simulink subsystem "ButtonStatesRF"  (R2023b) (continued)
== stateflow chart ButtonStatesRF/ButtonStates/buttonFlowChart == (continued)
                  Flow.ButtonTriggeredMenu.Menu.MainSubMenu.SettingsSubMenu.ClockSubMenu.DateSubMenu.DateYYYYMMDD.DateDigit3 -> Flow.ButtonTriggeredMenu.Menu.MainSubMenu.SettingsSubMenu.ClockSubMenu.DateSubMenu.DateYYYYMMDD.DateDigit2 [order 1] : EXIT
                  (junction 7832) -> 7840
                  (junction 7837) -> (junction 7832) : {BLU_FnClkSaveDate();}
                  Flow.ButtonTriggeredMenu.Menu.MainSubMenu.SettingsSubMenu.ClockSubMenu.DateSubMenu.DateYYYYMMDD.DateDigit3 -> (junction 7833) [order 6] : longUP
                      | {BLU_FnClkIncDateDig1();}
                  (junction 7833) -> (junction 7834)
                  Flow.ButtonTriggeredMenu.Menu.MainSubMenu.SettingsSubMenu.ClockSubMenu.DateSubMenu.DateYYYYMMDD.DateDigit3 -> (junction 7834) [order 4] : UP
                      | {BLU_FnClkIncDateDig1();}
                  (junction 7834) -> (junction 7835)
                  Flow.ButtonTriggeredMenu.Menu.MainSubMenu.SettingsSubMenu.ClockSubMenu.DateSubMenu.DateYYYYMMDD.DateDigit3 -> (junction 7835) [order 3] : DOWN
                      | {BLU_FnClkDecDateDig1();}
                  (junction 7835) -> (junction 7836)
                  Flow.ButtonTriggeredMenu.Menu.MainSubMenu.SettingsSubMenu.ClockSubMenu.DateSubMenu.DateYYYYMMDD.DateDigit3 -> (junction 7836) [order 2] : longDOWN
                      | {BLU_FnClkDecDateDig1();}
                  (junction 7836) -> (junction 7838)
                  Flow.ButtonTriggeredMenu.Menu.MainSubMenu.SettingsSubMenu.ClockSubMenu.DateSubMenu.DateYYYYMMDD.DateDigit3 -> (junction 7837) [order 5] : SET
                  (junction 7838) -> Flow.ButtonTriggeredMenu.Menu.MainSubMenu.SettingsSubMenu.ClockSubMenu.DateSubMenu.DateYYYYMMDD.DateDigit3
                7679 -> (junction 7674)
                (junction 7674) -> 7680 [order 1] : [dateFormat == eStateName.DATE_FORMAT_DDMMYYYY]
                (junction 7674) -> (junction 7676) [order 2]
                7681 -> (junction 7675)
                (junction 7675) -> (junction 7677)
                (junction 7676) -> 7682 [order 1] : [dateFormat == eStateName.DATE_FORMAT_MMDDYYYY]
                (junction 7676) -> (junction 7678) [order 2]
                7683 -> (junction 7677)
                (junction 7677) -> 7686
                (junction 7678) -> 7684
                7685 -> 7686
              state SetClockFormat
                (history)
                state SetClockFormatMenu
                  state Clock12H
                    en:
                    currentState = eStateName.CLOCK_FORMAT_12H;
                  state Clock24H
                    en:
                    currentState = eStateName.CLOCK_FORMAT_24H;
                  Clock12H -> Clock24H : UP || DOWN
                  Clock24H -> Clock12H : UP || DOWN
                7884 -> Clock12H
                SetClockFormatMenu -> 7885 [order 1] : EXIT
                (junction 7883) -> 7885
                SetClockFormatMenu -> (junction 7883) [order 2] : SET
                    | {BLU_FnClkFrmtTgle(currentState);}
              state SetDateFormat
                (history)
                state SetDateFormatMenu
                  (history)
                  state DateFormat1
                    en:
                    currentState = eStateName.DATE_FORMAT_DDMMYYYY;
                  state DateFormat2
                    en:
                    currentState = eStateName.DATE_FORMAT_MMDDYYYY;
                  state DateFormat3
                    en:
                    currentState = eStateName.DATE_FORMAT_YYYYMMDD;
                  (default) -> DateFormat1
                  (junction 7905) -> DateFormat1
                  DateFormat1 -> (junction 7906) [order 2] : UP
                  (junction 7907) -> (junction 7905)
                  (junction 7906) -> (junction 7908)
                  DateFormat1 -> DateFormat2 [order 1] : DOWN
                  DateFormat2 -> DateFormat1 [order 2] : UP
                  DateFormat2 -> DateFormat3 [order 1] : DOWN
                  DateFormat3 -> DateFormat2 [order 2] : UP
                  DateFormat3 -> (junction 7907) [order 1] : DOWN
                  (junction 7908) -> DateFormat3
                7897 -> DateFormat1
                SetDateFormatMenu -> 7898 [order 1] : EXIT
                (junction 7896) -> 7898 : {dateFormat =currentState;
                    | BLU_FnDateFrmtTgle(currentState);}
                SetDateFormatMenu -> (junction 7896) [order 2] : SET
              (default) -> ClockDateMenu
              7582 -> (junction 7612)
              (junction 7613) -> 7583 [order 1] : SET
              7584 -> (junction 7577)
              (junction 7577) -> (junction 7578)
              (junction 7579) -> 7585
              Date -> 7586 [order 3] : SET
              7587 -> (junction 7578)
              ClockDateMenu -> (junction 7579) : EXIT
              (junction 7578) -> (junction 7580)
              (junction 7579) -> 7588 [commented out] : [IgnBattFlg==true]
              ClockFormat -> 7589 [order 3] : SET
              7590 -> (junction 7580)
              (junction 7580) -> (junction 7581)
              DateFormat -> 7591 [order 3] : SET
              (junction 7581) -> ClockDateMenu
              7592 -> (junction 7581)
            state UnitsSubMenu
              (history)
              state UnitsMenu
                (history)
                state Distance
                  en:
                  currentState = eStateName.DISTANCE;
                state Temperature
                  en:
                  currentState = eStateName.TEMPERATURE;
                state Consumption
                  en:
                  currentState = eStateName.CONSUMPTION;
                (default) -> Distance
                (junction 7942) -> Distance
                (junction 7945) -> (junction 7942)
                Distance -> (junction 7943) [order 3] : UP
                (junction 7943) -> (junction 7944)
                Distance -> Temperature [order 1] : DOWN
                Temperature -> Distance [order 1] : UP
                Temperature -> Consumption [order 2] : DOWN
                Consumption -> Temperature [order 1] : UP
                (junction 7944) -> Consumption
                Consumption -> (junction 7945) [order 3] : DOWN
              state DistanceSubMenu
                state DstnceMenu
                  (history)
                  state Kilometer
                    en:
                    currentState = eStateName.DISTANCE_KILOMETER;
                  state Miles
                    en:
                    currentState = eStateName.DISTANCE_MILES;
                  (default) -> Kilometer
                  Kilometer -> Miles : DOWN || UP
                  Miles -> Kilometer : UP || DOWN
                7962 -> DstnceMenu
                DstnceMenu -> 7963 [order 1] : EXIT
                (junction 7961) -> 7963 : {BLU_FnSETDistUnits(currentState);}
                DstnceMenu -> (junction 7961) [order 2] : SET
              state TemperatureSubMenu
                state TempUnitsMenu
                  (history)
                  state Celsius
                    en:
                    currentState = eStateName.TEMPERATURE_CELSIUS;
                  state Farenheit
                    en:
                    currentState = eStateName.TEMPERATURE_FARENHEIT;
                  (default) -> Celsius
                  Celsius -> Farenheit : DOWN || UP
                  Farenheit -> Celsius : UP || DOWN
                7977 -> TempUnitsMenu
                TempUnitsMenu -> (junction 7976) [order 2] : SET
                (junction 7976) -> 7978 : {BLU_FnSETTempUnits(currentState);}
                TempUnitsMenu -> 7978 [order 1] : EXIT
              state ConsumptionSubMenu
                state ConsUnits
                  (history)
                  state litres_100km
                    en:
                    currentState = eStateName.CONSUMPTION_LTR_PER_100KM;
                  state km_l
                    en:
                    currentState = eStateName.CONSUMPTION_KM_PER_LTR;
                  state USg_100mile
                    en:
                    currentState = eStateName.CONSUMPTION_USG_PER_100MI;
                  state Mi_USg
                    en:
                    currentState = eStateName.CONSUMPTION_MI_PER_USG;
                  state Mi_Litre
                    en:
                    currentState = eStateName.CONSUMPTION_MI_PER_LTR;
                  state UKg_100Mi
                    en:
                    currentState = eStateName.CONSUMPTION_UKG_PER_100MI;
                  state Mi_UKg
                    en:
                    currentState = eStateName.CONSUMPTION_MI_PER_UKG;
                  state Li_100Mi
                    en:
                    currentState = eStateName.CONSUMPTION_L_PER_100MI;
                  (default) -> litres_100km
                  litres_100km -> (junction 8000) [order 2] : UP
                  (junction 8001) -> litres_100km
                  (junction 8000) -> (junction 8003)
                  (junction 8002) -> (junction 8001)
                  litres_100km -> km_l [order 1] : DOWN
                  km_l -> litres_100km [order 1] : UP
                  km_l -> USg_100mile [order 2] : DOWN
                  USg_100mile -> km_l [order 1] : UP
                  USg_100mile -> Mi_USg [order 2] : DOWN
                  Mi_USg -> USg_100mile [order 1] : UP
                  Mi_USg -> Mi_Litre [order 2] : DOWN
                  Mi_Litre -> Mi_USg [order 1] : UP
                  Mi_Litre -> UKg_100Mi [order 2] : DOWN
                  UKg_100Mi -> Mi_Litre [order 1] : UP
                  UKg_100Mi -> Mi_UKg [order 2] : DOWN
                  Mi_UKg -> UKg_100Mi [order 1] : UP
                  Mi_UKg -> Li_100Mi [order 2] : DOWN
                  Li_100Mi -> Mi_UKg [order 1] : UP
                  Li_100Mi -> (junction 8002) [order 2] : DOWN
                  (junction 8003) -> Li_100Mi
                7992 -> ConsUnits
                ConsUnits -> 7993 [order 1] : EXIT
                (junction 7991) -> 7993 : {BLU_FnSETConsUnits(currentState);}
                ConsUnits -> (junction 7991) [order 2] : SET
              7924 -> UnitsMenu
              Distance -> 7925 [order 2] : SET
              7926 -> UnitsMenu
              UnitsMenu -> 7927 : EXIT
              Temperature -> 7928 [order 3] : SET
              7929 -> UnitsMenu
              Consumption -> 7930 [order 2] : SET
              7931 -> UnitsMenu
            state LanguageSubMenu
              (history)
              state LanguageSetSubMenu
                (history)
                state EnglishUS
                  en:
                  currentState = eStateName.ENGLISH_US;
                state EnglishUK
                  en:
                  currentState = eStateName.ENGLISH_UK;
                state German
                  en:
                  currentState = eStateName.GERMAN;
                state Italian
                  en:
                  currentState = eStateName.ITALIAN;
                state French
                  en:
                  currentState = eStateName.FRENCH;
                state Spanish
                  en:
                  currentState = eStateName.SPANISH;
                (junction 8043) -> EnglishUS
                (junction 8046) -> (junction 8043)
                EnglishUS -> (junction 8044) [order 2] : UP
                (junction 8044) -> (junction 8045)
                EnglishUS -> EnglishUK [order 1] : DOWN
                EnglishUK -> EnglishUS [order 1] : UP
                EnglishUK -> German [order 2] : DOWN
                German -> EnglishUK [order 1] : UP
                German -> Italian [order 2] : DOWN
                Italian -> German [order 1] : UP
                Italian -> French [order 2] : DOWN
                French -> Italian [order 1] : UP
                French -> Spanish [order 2] : DOWN
                Spanish -> French [order 1] : UP
                (junction 8045) -> Spanish
                Spanish -> (junction 8046) [order 2] : DOWN
              8035 -> (junction 8043)
              LanguageSetSubMenu -> 8036 [order 1] : EXIT
              (junction 8034) -> 8036 : {BLU_FnSETLanguage(currentState);}
              LanguageSetSubMenu -> (junction 8034) [order 2] : SET
            state HeatingGripsSettingsSubMenu
              (history)
              state HtdGripsSel
                (history)
                state HeatingGripsNotAvailable
                  en:
                  currentState = eStateName.HEATING_GRIPS_NOT_AVAILABLE;
                state HeatingGripsAvailable
                  en:
                  currentState = eStateName.HEATING_GRIPS_AVAILABLE;
                HeatingGripsNotAvailable -> HeatingGripsAvailable [order 1] : DOWN || UP
                HeatingGripsAvailable -> HeatingGripsNotAvailable [order 1] : DOWN || UP
                HeatingGripsAvailable -> HeatingGripsAvailable [commented out] : SET
                    | {heatedGrips = eFeatureStatus.available;
                    | BLU_FnSetHeatedGrips(heatedGrips);}
                    | //TBD Heated_Grips variable set
              state HCU_GripHeat_LvlChk
                en:
                currentState = eStateName.HCU_GRIP_HEAT_LVL_CHK;
              HeatingGripsNotAvailable -> 8072 [order 2] : SET
                  | {heatedGrips = eFeatureStatus.notAvailable;
                  | DASH_Grip_Installation = 0;
                  | BLU_FnSetHeatedGrips(heatedGrips);}
                  | //TBD Heated_Grips variable set
              (junction 8070) -> 8072
              8073 -> HeatingGripsNotAvailable
              HtdGripsSel -> (junction 8070) : EXIT
              (junction 8071) -> (junction 8070) : [HCU_Grip_Heat_Level_Res ==0 || after(3,sec)]
              HCU_GripHeat_LvlChk -> (junction 8070) [order 1] : [HCU_Grip_Heat_Level_Res !=0]
                  | {heatedGrips = eFeatureStatus.notAvailable;}
              HCU_GripHeat_LvlChk -> (junction 8071) [order 2]
              HeatingGripsAvailable -> HCU_GripHeat_LvlChk [order 2] : SET
                  | {heatedGrips = eFeatureStatus.available;
                  | DASH_Grip_Installation = 1;}
                  | //TBD Heated_Grips variable set
            state ExtraFunctionsSubMenu
              %en:
              %currentState = eStateName.EXTRA_FUNCTIONS_SUB_MN;
              state ExtraFncSubMenu
                state ExtraFnCall
                  en:
                  currentState = eStateName.EXTRA_FN_CALL;
                state ExtraFnAudio
                  en:
                  currentState = eStateName.EXTRA_FN_AUDIO;
                state ExtraFnNavigation
                  en:
                  currentState = eStateName.EXTRA_FN_NAVIGATION;
                state ExtraFnRemoteCtrl
                  en:
                  currentState = eStateName.EXTRA_FN_REMOTE_CTRL;
                (default) -> Flow.ButtonTriggeredMenu.Menu.MainSubMenu.SettingsSubMenu.ExtraFunctionsSubMenu.ExtraFncSubMenu.ExtraFnCall
                Flow.ButtonTriggeredMenu.Menu.MainSubMenu.SettingsSubMenu.ExtraFunctionsSubMenu.ExtraFncSubMenu.ExtraFnCall -> 8337 [order 3] : SET
                (junction 8333) -> Flow.ButtonTriggeredMenu.Menu.MainSubMenu.SettingsSubMenu.ExtraFunctionsSubMenu.ExtraFncSubMenu.ExtraFnCall
                (junction 8331) -> (junction 8333)
                Flow.ButtonTriggeredMenu.Menu.MainSubMenu.SettingsSubMenu.ExtraFunctionsSubMenu.ExtraFncSubMenu.ExtraFnCall -> (junction 8324) [order 2] : UP
                (junction 8324) -> (junction 8326)
                Flow.ButtonTriggeredMenu.Menu.MainSubMenu.SettingsSubMenu.ExtraFunctionsSubMenu.ExtraFncSubMenu.ExtraFnCall -> Flow.ButtonTriggeredMenu.Menu.MainSubMenu.SettingsSubMenu.ExtraFunctionsSubMenu.ExtraFncSubMenu.ExtraFnAudio [order 1] : DOWN
                Flow.ButtonTriggeredMenu.Menu.MainSubMenu.SettingsSubMenu.ExtraFunctionsSubMenu.ExtraFncSubMenu.ExtraFnAudio -> Flow.ButtonTriggeredMenu.Menu.MainSubMenu.SettingsSubMenu.ExtraFunctionsSubMenu.ExtraFncSubMenu.ExtraFnCall [order 1] : UP
                Flow.ButtonTriggeredMenu.Menu.MainSubMenu.SettingsSubMenu.ExtraFunctionsSubMenu.ExtraFncSubMenu.ExtraFnAudio -> 8340 [order 3] : SET
                Flow.ButtonTriggeredMenu.Menu.MainSubMenu.SettingsSubMenu.ExtraFunctionsSubMenu.ExtraFncSubMenu.ExtraFnAudio -> Flow.ButtonTriggeredMenu.Menu.MainSubMenu.SettingsSubMenu.ExtraFunctionsSubMenu.ExtraFncSubMenu.ExtraFnNavigation [order 2] : DOWN
                Flow.ButtonTriggeredMenu.Menu.MainSubMenu.SettingsSubMenu.ExtraFunctionsSubMenu.ExtraFncSubMenu.ExtraFnNavigation -> Flow.ButtonTriggeredMenu.Menu.MainSubMenu.SettingsSubMenu.ExtraFunctionsSubMenu.ExtraFncSubMenu.ExtraFnAudio [order 1] : UP
                Flow.ButtonTriggeredMenu.Menu.MainSubMenu.SettingsSubMenu.ExtraFunctionsSubMenu.ExtraFncSubMenu.ExtraFnNavigation -> 8343 [order 3] : SET
                Flow.ButtonTriggeredMenu.Menu.MainSubMenu.SettingsSubMenu.ExtraFunctionsSubMenu.ExtraFncSubMenu.ExtraFnNavigation -> Flow.ButtonTriggeredMenu.Menu.MainSubMenu.SettingsSubMenu.ExtraFunctionsSubMenu.ExtraFncSubMenu.ExtraFnRemoteCtrl [order 2] : DOWN
                Flow.ButtonTriggeredMenu.Menu.MainSubMenu.SettingsSubMenu.ExtraFunctionsSubMenu.ExtraFncSubMenu.ExtraFnRemoteCtrl -> Flow.ButtonTriggeredMenu.Menu.MainSubMenu.SettingsSubMenu.ExtraFunctionsSubMenu.ExtraFncSubMenu.ExtraFnNavigation [order 1] : UP
                (junction 8326) -> Flow.ButtonTriggeredMenu.Menu.MainSubMenu.SettingsSubMenu.ExtraFunctionsSubMenu.ExtraFncSubMenu.ExtraFnRemoteCtrl
                Flow.ButtonTriggeredMenu.Menu.MainSubMenu.SettingsSubMenu.ExtraFunctionsSubMenu.ExtraFncSubMenu.ExtraFnRemoteCtrl -> 8345 [order 3] : SET
                Flow.ButtonTriggeredMenu.Menu.MainSubMenu.SettingsSubMenu.ExtraFunctionsSubMenu.ExtraFncSubMenu.ExtraFnRemoteCtrl -> (junction 8331) [order 2] : DOWN
              state ExtraFnCall
                en:
                currentState = eStateName.EXTRA_FN_CALL_WAIT;
              state ExtraFnAudio
                en:
                currentState = eStateName.EXTRA_FN_AUDIO_WAIT;
              state ExtraFnNavigation
                en:
                currentState = eStateName.EXTRA_FN_NAVIGATION_WAIT;
              state ExtraFnRemoteCtrl
                en:
                currentState = eStateName.EXTRA_FN_REMOTE_CTRL_WAIT;
              (default) -> ExtraFncSubMenu
              8338 -> Flow.ButtonTriggeredMenu.Menu.MainSubMenu.SettingsSubMenu.ExtraFunctionsSubMenu.ExtraFnCall
              Flow.ButtonTriggeredMenu.Menu.MainSubMenu.SettingsSubMenu.ExtraFunctionsSubMenu.ExtraFnCall -> (junction 8359)
              (junction 8359) -> (junction 8361)
              8342 -> Flow.ButtonTriggeredMenu.Menu.MainSubMenu.SettingsSubMenu.ExtraFunctionsSubMenu.ExtraFnAudio
              Flow.ButtonTriggeredMenu.Menu.MainSubMenu.SettingsSubMenu.ExtraFunctionsSubMenu.ExtraFnAudio -> (junction 8361)
              (junction 8361) -> (junction 8364)
              8347 -> Flow.ButtonTriggeredMenu.Menu.MainSubMenu.SettingsSubMenu.ExtraFunctionsSubMenu.ExtraFnNavigation
              Flow.ButtonTriggeredMenu.Menu.MainSubMenu.SettingsSubMenu.ExtraFunctionsSubMenu.ExtraFnNavigation -> (junction 8364)
              (junction 8364) -> (junction 8367)
              8348 -> Flow.ButtonTriggeredMenu.Menu.MainSubMenu.SettingsSubMenu.ExtraFunctionsSubMenu.ExtraFnRemoteCtrl
              Flow.ButtonTriggeredMenu.Menu.MainSubMenu.SettingsSubMenu.ExtraFunctionsSubMenu.ExtraFnRemoteCtrl -> (junction 8367)
              (junction 8367) -> (junction 8370)
              (junction 8370) -> ExtraFncSubMenu : [after(BLU_ExtraFnWtTmr_Tms,msec)]
              ExtraFncSubMenu -> 8375 : EXIT
            6185 -> 6186
            6187 -> 6188
            6189 -> (junction 6255)
            6191 -> 6190
            6192 -> 6193
            SettingsMenuScreens -> 6194 : EXIT
            6196 -> 6195
            6197 -> 6198
            6199 -> 6200
            6202 -> 6201
            6203 -> 6204
            6206 -> 6205
            6207 -> ClockSubMenu
            6208 -> 6211 [commented out]
            6209 -> 6210
            6213 -> 6212
            6214 -> 6215
            6217 -> 6216
            6218 -> 6219
            6221 -> 6220
            6222 -> 6223
            6225 -> 6224
            6226 -> ExtraFunctionsSubMenu
            8376 -> 6227
          state SetService
            (history)
            state ServiceMenu
              (history)
              state SetServiceDistance
                state SetServiceDistanceKm
                  en:
                  currentState = eStateName.SET_SERVICE_DISTANCE_KM;
                state SetServiceDistanceMi
                  en:
                  currentState = eStateName.SET_SERVICE_DISTANCE_MI;
                (default) -> (junction 8152)
                (junction 8152) -> Flow.ButtonTriggeredMenu.Menu.MainSubMenu.SetService.ServiceMenu.SetServiceDistance.SetServiceDistanceKm [order 1] : [odoUnits == 0]
                (junction 8152) -> (junction 8153) [order 2]
                (junction 8153) -> Flow.ButtonTriggeredMenu.Menu.MainSubMenu.SetService.ServiceMenu.SetServiceDistance.SetServiceDistanceMi
              state SetServiceMonths
                en:
                currentState = eStateName.SET_SERVICE_MONTHS_MM;
              state SoftwareVersion
                en:
                currentState = eStateName.SET_SERVICE_SOFTWARE_VERSION;
              (default) -> SetServiceDistance
              SetServiceDistance -> (junction 8385) [order 3] : UP
              (junction 8385) -> (junction 8387)
              (junction 8392) -> SetServiceDistance
              (junction 8397) -> (junction 8392)
              SetServiceDistance -> SetServiceMonths [order 2] : DOWN
              SetServiceMonths -> SetServiceDistance [order 2] : UP
              SetServiceMonths -> SoftwareVersion [order 3] : DOWN
              SoftwareVersion -> SetServiceMonths [order 1] : UP
              (junction 8387) -> SoftwareVersion
              SoftwareVersion -> (junction 8397) [order 2] : DOWN
            state SetServiceDistanceMenu
              (history)
              state DistanceModifyMenu
                (history)
                state SetServiceDistanceKm
                  en:
                  currentState = eStateName.CURSOR_ON_KM;
                state SetServiceDistanceMi
                  en:
                  currentState = eStateName.CURSOR_ON_MI;
              (default) -> (junction 8163)
              Flow.ButtonTriggeredMenu.Menu.MainSubMenu.SetService.SetServiceDistanceMenu.DistanceModifyMenu.SetServiceDistanceKm -> (junction 8162) [order 2] : DOWN
              (junction 8162) -> (junction 8166) : {BLU_FnDecSrvcDistKM();}
              (junction 8163) -> Flow.ButtonTriggeredMenu.Menu.MainSubMenu.SetService.SetServiceDistanceMenu.DistanceModifyMenu.SetServiceDistanceKm [order 1] : [odoUnits == 0]
              Flow.ButtonTriggeredMenu.Menu.MainSubMenu.SetService.SetServiceDistanceMenu.DistanceModifyMenu.SetServiceDistanceKm -> (junction 8164) [order 1] : UP
              (junction 8163) -> (junction 8168) [order 2]
              (junction 8164) -> (junction 8165) : {BLU_FnIncSrvcDistKM();}
              (junction 8165) -> DistanceModifyMenu
              (junction 8166) -> (junction 8165)
              (junction 8167) -> (junction 8165) : {BLU_FnIncSrvcDistMI();}
              (junction 8169) -> (junction 8166) : {BLU_FnDecSrvcDistMI();}
              Flow.ButtonTriggeredMenu.Menu.MainSubMenu.SetService.SetServiceDistanceMenu.DistanceModifyMenu.SetServiceDistanceMi -> (junction 8167) [order 1] : UP
              (junction 8168) -> Flow.ButtonTriggeredMenu.Menu.MainSubMenu.SetService.SetServiceDistanceMenu.DistanceModifyMenu.SetServiceDistanceMi
              Flow.ButtonTriggeredMenu.Menu.MainSubMenu.SetService.SetServiceDistanceMenu.DistanceModifyMenu.SetServiceDistanceMi -> (junction 8169) [order 2] : DOWN
            state SetServiceMonthsMenu
              en:
              currentState = eStateName.CURSON_ON_DATE;
            (default) -> ServiceMenu
            ServiceMenu -> (junction 8123) [order 2] : EXIT
            (junction 8123) -> 8129
            ServiceMenu -> 8129 [order 1] : [duration(set,msec) >=3000]
                | {BLU_FnSaveSrvcChanges();}
            SetServiceDistance -> SetServiceDistanceMenu [order 1] : SET
            SetServiceDistanceMenu -> (junction 8124) : EXIT || SET
            (junction 8124) -> ServiceMenu
            SetServiceMonthsMenu -> (junction 8124) [order 3] : EXIT || SET
            SetServiceMonthsMenu -> (junction 8125) [order 1] : UP
            (junction 8125) -> (junction 8126) : {BLU_FnIncSrvcMonths();}
            SetServiceMonths -> SetServiceMonthsMenu [order 1] : SET
            (junction 8126) -> SetServiceMonthsMenu
            (junction 8128) -> (junction 8126)
            SetServiceMonthsMenu -> (junction 8127) [order 2] : DOWN
            (junction 8127) -> (junction 8128) : {BLU_FnDecSrvcMonths();}
          (default) -> (junction 3141)
          (junction 8255) -> 8260 [order 2] : EXIT
          5276 -> (junction 3141)
          (junction 3141) -> LapTimer [order 1] : [rideMode == eRideMode.track && ...
              | variant !=eBikeVariant.duke200]
          (junction 3141) -> Motorcycle [order 2]
          (junction 3145) -> (junction 3141)
          SettingsMenu -> (junction 8193) [order 2]
          (junction 8255) -> 8199 [order 1] : SET
          (junction 8091) -> (junction 8255) [order 2]
          LapTimer -> (junction 3151) [order 2] : UP
          8198 -> LapTimer
          (junction 4100) -> (junction 3151) [order 2]
          (junction 3151) -> (junction 3153)
          (junction 4100) -> LapTimer [order 1] : [rideMode == eRideMode.track && ...
              | variant !=eBikeVariant.duke200]
          LapTimer -> Motorcycle [order 1] : DOWN
          LapTimerEntry -> (junction 8255)
          Motorcycle -> (junction 4100) [order 1] : UP
          NavigationVisible -> (junction 8100)
          5941 -> 5671
          Motorcycle -> (junction 8091) [order 3]
          (junction 8091) -> 5672 [order 1] : SET
          (junction 8093) -> (junction 8091) [order 2]
          5944 -> 5670
          5673 -> Motorcycle
          5947 -> 5669
          Motorcycle -> BikeInfo [order 2] : DOWN
          BikeInfo -> Motorcycle [order 1] : UP
          5568 -> 5520
          BikeInfo -> (junction 8093) [order 3]
          (junction 8093) -> 5518 [order 1] : SET
          (junction 8095) -> (junction 8093) [order 2]
          5519 -> BikeInfo
          BikeInfo -> TripInfo [order 2] : DOWN
          TripInfo -> BikeInfo [order 1] : UP
          AudioVisible -> (junction 8107)
          (junction 8095) -> 5573 [order 1] : SET
          TripInfo -> (junction 8095) [order 3]
          5664 -> 5570
          (junction 8097) -> (junction 8095) [order 2]
          (junction 839) -> TripInfo
          5572 -> (junction 839)
          5666 -> 5571
          CruiseControlMain -> (junction 839) [order 1] : UP
          (junction 194) -> (junction 839) [order 2]
          TripInfo -> (junction 154) [order 2] : DOWN
          6030 -> 5950
          CruiseControlMain -> (junction 8097) [order 3]
          (junction 8097) -> 5952 [order 1] : SET
          (junction 8100) -> (junction 8097) [order 2]
          (junction 154) -> CruiseControlMain [order 1] : [variant !=eBikeVariant.duke200 && ...
              | (ECU_SL_Feature == 1 || ...
              | rideMode != eRideMode.track)]
          5951 -> (junction 194)
          (junction 194) -> CruiseControlMain [order 1] : [ECU_SL_Feature == 1 && ...
              | variant !=eBikeVariant.duke200]
          NavigationMain -> (junction 194) [order 2] : UP
          (junction 154) -> (junction 157) [order 2]
          (junction 189) -> (junction 194) [order 2]
          CruiseControlMain -> (junction 157) [order 2] : DOWN
          6085 -> 6033
          (junction 8100) -> 6035 [order 1] : SET
          (junction 8103) -> (junction 8100) [order 2]
          (junction 157) -> NavigationMain [order 1] : [CCU_Status == eCCUStatus.installed &&...
              |  CCU_TbT_Navigation_Feature == 1 &&...
              |  btPhonePrngScs ]
          (junction 189) -> NavigationMain [order 1] : [CCU_Status == eCCUStatus.installed &&...
              |  CCU_TbT_Navigation_Feature == 1 &&...
              | btPhonePrngScs]
          6034 -> (junction 189)
          (junction 157) -> (junction 163) [order 2]
          HeatedGripsMain -> (junction 189) [order 2] : UP
          (junction 186) -> (junction 189) [order 2]
          NavigationMain -> (junction 163) [order 1] : DOWN
          HeatedGripsMain -> (junction 8103) [order 3]
          (junction 8103) -> 6089 [order 1] : SET
          6123 -> 6088
          (junction 8107) -> (junction 8103) [order 2]
          (junction 163) -> HeatedGripsMain [order 1] : [variant !=eBikeVariant.duke200 && ...
              | Heated_Grips == 1 ]
              |  //Menu Settings/Heating/Heating Grip == Not available]
          (junction 163) -> (junction 171) [order 2]
          (junction 186) -> HeatedGripsMain [order 1] : [Heated_Grips == 1 &&...
              | variant !=eBikeVariant.duke200 ]
          6090 -> (junction 186)
          Flow.ButtonTriggeredMenu.Menu.MainSubMenu.AudioMain -> (junction 186) [order 2] : UP
          (junction 183) -> (junction 186) [order 2] : %[CCU_Status == eCCUStatus.notInstalled ||...
              | % CCU_Music_Feature == 0]
          HeatedGripsMain -> (junction 171) [order 1] : DOWN
          6167 -> 6126
          (junction 8107) -> 6128 [order 1] : SET
          (junction 8193) -> (junction 8107) [order 2]
          (junction 171) -> Flow.ButtonTriggeredMenu.Menu.MainSubMenu.AudioMain [order 1] : [CCU_Status == eCCUStatus.installed &&...
              |  CCU_Music_Feature == 1 &&...
              | btPhonePrngScs]
          (junction 171) -> (junction 177) [order 2]
          6127 -> (junction 183)
          (junction 183) -> Flow.ButtonTriggeredMenu.Menu.MainSubMenu.AudioMain [order 1] : [CCU_Status == eCCUStatus.installed &&...
              |  CCU_Music_Feature == 1 &&...
              | btPhonePrngScs]
          (junction 5313) -> (junction 183)
          (junction 181) -> (junction 183)
          Flow.ButtonTriggeredMenu.Menu.MainSubMenu.AudioMain -> (junction 177) [order 1] : DOWN
          (junction 3153) -> (junction 4104)
          (junction 4104) -> (junction 5313) [order 2]
          (junction 8307) -> (junction 5313)
          (junction 4104) -> SettingsMain [order 1] : [vehSpeed<2.5]
          SettingsMain -> (junction 5313) [order 3] : [vehSpeed>3.0]
          SettingsSubMenu -> (junction 8307) : [vehSpeed>3.0]
          SettingsMain -> (junction 181) [order 1] : UP
          (junction 8193) -> 6179 [order 1] : SET
          8118 -> 6181
          (junction 177) -> SettingsMain [order 1] : [vehSpeed<=2.5]
          (junction 177) -> (junction 3145) [order 2]
          (junction 8189) -> SettingsMain
          6180 -> (junction 8189)
          8121 -> (junction 8189)
          SettingsMain -> (junction 3145) [order 2] : DOWN
          3012 -> SetService
        state Lap_TimerV1 [commented out]
          state LapTimerLoop [commented out]
            en:
            currentState = eStateName.RESET_LAPS;
            roundTimer = roundTimerData_arr[roundTimeCounter];
          state ResetLaps [commented out]
            state Keep [commented out]
              en:
              currentState = eStateName.KEEP;
            state Reset [commented out]
              en:
              currentState = eStateName.RESET;
              roundTimerData_arr[roundTimeCounter] =0;
              //updateRoundTimeData();
            (default) -> Flow.ButtonTriggeredMenu.Menu.Lap_TimerV1.ResetLaps.Keep [commented out]
            Flow.ButtonTriggeredMenu.Menu.Lap_TimerV1.ResetLaps.Keep -> Flow.ButtonTriggeredMenu.Menu.Lap_TimerV1.ResetLaps.Reset [commented out] : DOWN
            Flow.ButtonTriggeredMenu.Menu.Lap_TimerV1.ResetLaps.Reset -> Flow.ButtonTriggeredMenu.Menu.Lap_TimerV1.ResetLaps.Keep [commented out] : UP
          (default) -> Flow.ButtonTriggeredMenu.Menu.Lap_TimerV1.LapTimerLoop [commented out] : {roundTimeCounter = 0;
              | copyRoundTimeData();
              | updateRoundTimeData();}
          Flow.ButtonTriggeredMenu.Menu.Lap_TimerV1.LapTimerLoop -> (junction 237) [commented out] : DOWN
          (junction 237) -> (junction 240) [commented out] : [roundTimeCounter < BLU_MaxRndTimeLoopLim]
          (junction 240) -> (junction 239) [commented out] : {roundTimeCounter++;}
          (junction 237) -> (junction 239) [commented out]
          (junction 239) -> Flow.ButtonTriggeredMenu.Menu.Lap_TimerV1.LapTimerLoop [commented out]
          Flow.ButtonTriggeredMenu.Menu.Lap_TimerV1.LapTimerLoop -> (junction 245) [commented out] : UP
          (junction 245) -> (junction 247) [commented out] : [roundTimeCounter>0]
          (junction 247) -> (junction 249) [commented out] : {roundTimeCounter--;}
          (junction 245) -> (junction 249) [commented out]
          (junction 249) -> Flow.ButtonTriggeredMenu.Menu.Lap_TimerV1.LapTimerLoop [commented out]
          Flow.ButtonTriggeredMenu.Menu.Lap_TimerV1.LapTimerLoop -> Flow.ButtonTriggeredMenu.Menu.Lap_TimerV1.ResetLaps [commented out] : SET
          Flow.ButtonTriggeredMenu.Menu.Lap_TimerV1.ResetLaps -> Flow.ButtonTriggeredMenu.Menu.Lap_TimerV1.LapTimerLoop [commented out] : EXIT
        state NoQS
          en:
          QuickSelectorDbg = 1;
        (junction 2598) -> 2564
        4635 -> 2564
        NoQS -> (junction 2598) : [after(2,sec)]
        (junction 2147) -> (junction 2234) [order 1] : [quickSelectorUp ==eStateName.QS_UP_RIDE_MODE && ...
            | variant !=eBikeVariant.duke200]
        (junction 2147) -> NoQS [order 2]
        (junction 2425) -> NoQS
        (junction 2234) -> (junction 2233)
        (junction 2151) -> (junction 2147) [order 2]
        (junction 2336) -> (junction 2425) [order 2]
        2561 -> 5277
        (junction 2151) -> (junction 2238) [order 1] : [quickSelectorUp ==eStateName.QS_UP_ABS_MODE ]
        (junction 2238) -> (junction 2239)
        (junction 2168) -> (junction 2151) [order 2]
        (junction 2168) -> (junction 2243) [order 1] : [quickSelectorUp ==eStateName.QS_UP_MTC && ...
            | variant !=eBikeVariant.duke200 ]
        (junction 2243) -> (junction 2244)
        (junction 4035) -> (junction 2168) [order 2]
        (junction 2233) -> 5942
        (junction 2336) -> (junction 2233) [order 1] : [quickSelectorDown ==eStateName.QS_DOWN_RIDE_MODE && ...
            | variant !=eBikeVariant.duke200]
        (junction 2344) -> (junction 2336) [order 2]
        (junction 2239) -> 5946
        (junction 2344) -> (junction 2239) [order 1] : [quickSelectorDown ==eStateName.QS_DOWN_ABS_MODE ]
        (junction 2347) -> (junction 2344) [order 2]
        (junction 2244) -> 5949
        (junction 2347) -> (junction 2244) [order 1] : [quickSelectorDown ==eStateName.QS_DOWN_MTC && ...
            | variant !=eBikeVariant.duke200 ]
        (junction 4038) -> (junction 2347) [order 2]
        (junction 4035) -> (junction 4042) [order 1] : [quickSelectorUp ==eStateName.QS_UP_BIKEINFO ]
        (junction 4042) -> (junction 4037)
        (junction 2182) -> (junction 4035) [order 2]
        (junction 4037) -> 4675
        (junction 4038) -> (junction 4037) [order 1] : [quickSelectorDown ==eStateName.QS_DOWN_BIKEINFO ]
        (junction 2350) -> (junction 4038) [order 2]
        (junction 2182) -> (junction 2248) [order 1] : [quickSelectorUp ==eStateName.QS_UP_TRIP1 ]
        (junction 2248) -> (junction 2249)
        (junction 2216) -> (junction 2182) [order 2]
        (junction 2216) -> (junction 2253) [order 1] : [quickSelectorUp ==eStateName.QS_UP_TRIP2 ]
        (junction 2253) -> (junction 2254)
        (junction 2281) -> (junction 2216) [order 2]
        (junction 2249) -> 4647
        (junction 2350) -> (junction 2249) [order 1] : [quickSelectorDown ==eStateName.QS_DOWN_TRIP1 ]
        (junction 2353) -> (junction 2350) [order 2]
        (junction 2254) -> 5668
        (junction 2353) -> (junction 2254) [order 1] : [quickSelectorDown ==eStateName.QS_DOWN_TRIP2 ]
        (junction 2356) -> (junction 2353) [order 2]
        (junction 2281) -> (junction 2277) [order 1] : [quickSelectorUp ==eStateName.QS_UP_CRUISECONTROL && ...
            | variant !=eBikeVariant.duke200]
        (junction 2277) -> (junction 2278)
        (junction 2308) -> (junction 2281) [order 2]
        (junction 2278) -> 6032
        (junction 2356) -> (junction 2278) [order 1] : [quickSelectorDown ==eStateName.QS_DOWN_CRUISECONTROL && ...
            | variant !=eBikeVariant.duke200 ]
        (junction 2359) -> (junction 2356) [order 2]
        (junction 2308) -> (junction 2306) [order 1] : [quickSelectorUp ==eStateName.QS_UP_NAVIGATION ]
        (junction 2306) -> (junction 2304)
        (junction 2328) -> (junction 2308) [order 2]
        (junction 2359) -> (junction 2304) [order 1] : [quickSelectorDown ==eStateName.QS_DOWN_NAVIGATION ]
        (junction 2304) -> 6087
        (junction 2362) -> (junction 2359) [order 2]
        (junction 2328) -> (junction 2326) [order 1] : [quickSelectorUp ==eStateName.QS_UP_HEATEDGRIPS && ...
            | variant !=eBikeVariant.duke200 ]
        (junction 2326) -> (junction 2324)
        (junction 2226) -> (junction 2328) [order 2]
        (junction 2324) -> 6125
        (junction 2362) -> (junction 2324) [order 1] : [quickSelectorDown ==eStateName.QS_DOWN_HEATEDGRIPS  && ...
            | variant !=eBikeVariant.duke200]
        (junction 2365) -> (junction 2362) [order 2]
        (junction 2226) -> (junction 2262) [order 1] : [quickSelectorUp ==eStateName.QS_UP_AUDIO ]
        (junction 2262) -> (junction 2263)
        2569 -> (junction 2226)
        (junction 2263) -> 6169
        (junction 2365) -> (junction 2263) [order 1] : [quickSelectorDown ==eStateName.QS_DOWN_AUDIO ]
        2566 -> (junction 2365)
        4266 -> 8120
      4560 -> (junction 4862)
      (junction 5035) -> (junction 4862)
      (junction 4869) -> (junction 5035)
      (junction 4864) -> (junction 4869)
      (junction 4862) -> 2591
      Menu -> (junction 4869) [order 3] : RESET
      2565 -> (junction 4864)
      (default) -> MainScreen
      2594 -> 2562
      2603 -> 2570
      2606 -> 2567
      Menu -> MainScreen [order 1] : [hasChangedTo(INcall,true)]
      MainScreen -> (junction 4689)
      Menu -> (junction 4684) [order 2] : [hasChangedTo(IgnBattFlg,true)]
      (junction 4686) -> 4267
      (junction 4689) -> (junction 4686) : [hasChangedTo(IgnBattFlg,true)]
      (junction 4684) -> (junction 4686)
      4695 -> (junction 4686)
    state CheckBattIgn
      state WarningMenu
        state WarningInstruction
          en:
          popUpState = eStateName.POPUP_WARNING_INSTRUCTION;
        state HidePopUp
          en:
          popUpState = eStateName.POPUP_HIDE;
        (default) -> WarningInstruction
        WarningInstruction -> HidePopUp : UP || DOWN || SET || EXIT
        HidePopUp -> 3259 : after(0.1,sec)
      state CallOperator
        state ActiveCall
          en:
          currentState = eStateName.ACTIVE_CALL;
        state CallInstructionMenu
          en:
          popUpState = eStateName.POPUP_INCALL_INSTRUCTION;
        state AfterPhoneCall
          en:
          currentState = eStateName.AFTER_CALL;
        state hidePopUp
          en:
          popUpState = eStateName.POPUP_HIDE;
        (default) -> CallInstructionMenu
        ActiveCall -> (junction 3242) [order 2] : UP
            | {BLU_FnCallVolInc();}
        (junction 3242) -> (junction 3249)
        CallInstructionMenu -> ActiveCall [order 1] : [InCallStatus == 2]
        ActiveCall -> (junction 3249) [order 3] : DOWN
            | {BLU_FnCallVolDec();}
        (junction 3249) -> (junction 4209)
        CallInstructionMenu -> (junction 4215) [order 2] : [InCallStatus == 3]
        ActiveCall -> (junction 4215) [order 4] : [InCallStatus == 3]
        (junction 4215) -> AfterPhoneCall
        ActiveCall -> (junction 4209) [order 1] : EXIT
            | {BLU_FnActvCallEXIT();}
        (junction 4209) -> (junction 4212)
        CallInstructionMenu -> (junction 4201) [order 4] : EXIT
        (junction 4203) -> CallInstructionMenu
        CallInstructionMenu -> (junction 4202) [order 3] : SET
        ActiveCall -> (junction 4212) [order 5] : SET
            | {BLU_FnActvCallSET();}
        (junction 4201) -> (junction 4203) : {BLU_FnIncomingCallReject();}
        (junction 4202) -> (junction 4203) : {BLU_FnIncomingCallAnswer();}
        hidePopUp -> 3255 [order 1] : callEnded
        hidePopUp -> 3255 [order 2] : after(200,msec)
        AfterPhoneCall -> hidePopUp : after(4,sec)
    (default) -> ButtonTriggeredMenu
    ButtonTriggeredMenu -> WarningMenu [order 1] : warning
    3260 -> ButtonTriggeredMenu : {popUpState = eStateName.MAIN_SCREEN;}
    CheckBattIgn -> 4696 [order 1] : [hasChangedTo(IgnBattFlg,true)]
        | {BLU_FnClosePopUp();}
    ButtonTriggeredMenu -> CallOperator [order 2] : incomingCall
    3256 -> (junction 5326)
    (junction 5326) -> 4561 : {BLU_FnClosePopUp();}
    CheckBattIgn -> (junction 5326) [order 2] : RESET
  state PopUpChecker [parallel]
    settings: executionOrder=1
    state CheckWarning [parallel]
      settings: executionOrder=1
      state WarningDefault
      state WarningPopUp
        en:
        send(warning,Flow);
      (default) -> WarningDefault
      WarningDefault -> WarningPopUp : [hasChangedTo(INwarning,true)]
      WarningPopUp -> WarningDefault : after(100,msec)
    state CheckCall [parallel]
      settings: executionOrder=2
      state CallDefault
      state CallPopUp
        en:
      (default) -> CallDefault
      CallDefault -> CallPopUp : [hasChangedTo(INcall,true)]
      CallPopUp -> CallDefault : after(50,msec)
          | {send(incomingCall,Flow);}
    state CheckEndCall [parallel]
      settings: executionOrder=3
      state EndCallDefault
      state EndCallPopUp
        en:
        send(callEnded,Flow);
      (default) -> EndCallDefault
      EndCallDefault -> EndCallPopUp : [hasChangedTo(INcallEnded,true)]
      EndCallPopUp -> EndCallDefault : after(100,msec)
  state ButtonChecker [parallel]
    settings: executionOrder=2
    state CANnormal
      en:
      currentState = eStateName.CAN_NORMAL;
      state CheckUP [parallel]
        settings: executionOrder=1
        state upHigh
          en:
          DASH_RCM_Up_Switch = eButton.pressed;
          en,du:
          upDur = elapsed(sec);
        state upLow
          en:
          DASH_RCM_Up_Switch = eButton.released;
        state LongUPRpt
          en:
          send(longUP,Flow);
        (default) -> upLow
        upLow -> upHigh : [hasChangedTo(up,true)]
        (junction 1561) -> upLow
        (junction 1564) -> (junction 1561) : {send(UP, Flow);}
        (junction 1559) -> (junction 1564) [order 1] : [upDur*1000>=BLU_UpDtctMin_Tms && ...
            | upDur*1000 <=BLU_UpDtctMax_Tms ]
        upHigh -> (junction 1559) [order 1] : [hasChangedTo(up,false)]
        (junction 1576) -> (junction 1561)
        (junction 1559) -> (junction 1568) [order 2]
        (junction 1568) -> (junction 1577) [order 1] : [upDur*1000>=BLU_LngUpDtctMin_Tms && ...
            | upDur*1000 <=BLU_LngUpDtctMax_Tms ]
        (junction 1577) -> (junction 1576) : {send(longUP,Flow);}
        (junction 1568) -> (junction 1573) [order 2]
        (junction 1575) -> (junction 1576)
        upHigh -> LongUPRpt [order 2] : after(3,sec)
        (junction 1573) -> (junction 1575)
        (junction 5044) -> (junction 1575)
        LongUPRpt -> (junction 5044) [order 1] : [hasChangedTo(up,false)]
        LongUPRpt -> LongUPRpt [order 2] : [after(BLU_LngBtnEvntDur_Tms,msec)]
      state CheckDown [parallel]
        settings: executionOrder=2
        state downHigh
          en:
          DASH_RCM_Down_Switch = eButton.pressed;
          en,du:
          downDur = elapsed(sec);
        state downLow
          en:
          DASH_RCM_Down_Switch = eButton.released;
        state LongDownRpt
          en,du:
          send(longDOWN,Flow);
        (default) -> downLow
        downLow -> downHigh : [hasChangedTo(down,true)]
        (junction 1545) -> downLow
        (junction 1552) -> (junction 1545) : {send(DOWN, Flow);}
        (junction 1539) -> (junction 1552) [order 1] : [downDur*1000>=BLU_DwnDtctMin_Tms && ...
            | downDur*1000 <=BLU_DwnDtctMax_Tms ]
        downHigh -> (junction 1539) [order 1] : [hasChangedTo(down,false)]
        (junction 1556) -> (junction 1545)
        (junction 1539) -> (junction 1543) [order 2]
        (junction 1543) -> (junction 1542) [order 1] : [downDur*1000>=BLU_LngDwnDtctMin_Tms && ...
            | downDur*1000 <=BLU_LngDwnDtctMax_Tms ]
        (junction 1542) -> (junction 1556) : {send(longDOWN,Flow);}
        (junction 1543) -> (junction 1546) [order 2]
        (junction 1555) -> (junction 1556)
        downHigh -> LongDownRpt [order 2] : after(3,sec)
        (junction 1546) -> (junction 1555)
        (junction 5053) -> (junction 1555)
        LongDownRpt -> (junction 5053) [order 1] : [hasChangedTo(down,false)]
        LongDownRpt -> LongDownRpt [order 2] : [after(BLU_LngBtnEvntDur_Tms,msec)]
      state CheckSet [parallel]
        settings: executionOrder=3
        state setHigh
          en:
          DASH_RCM_Set_Switch = eButton.pressed;
          en,du:
          setDur = elapsed(sec);
        state setLow
          en:
          DASH_RCM_Set_Switch = eButton.released;
        state LongSETRpt
          en,du:
          send(longSET,Flow);
        (default) -> ButtonChecker.CANnormal.CheckSet.setLow
        ButtonChecker.CANnormal.CheckSet.setLow -> ButtonChecker.CANnormal.CheckSet.setHigh : [hasChangedTo(set,true)]
        (junction 1507) -> ButtonChecker.CANnormal.CheckSet.setLow
        (junction 1500) -> (junction 1507) : {send(SET,Flow);}
        (junction 1493) -> (junction 1500) [order 1] : [setDur*1000>=BLU_SetDtctMin_Tms && ...
            | setDur*1000 <=BLU_SetDtctMax_Tms ]
        ButtonChecker.CANnormal.CheckSet.setHigh -> (junction 1493) [order 1] : [hasChangedTo(set,false)]
        (junction 1508) -> (junction 1507)
        (junction 1493) -> (junction 1503) [order 2]
        (junction 1503) -> (junction 1505) [order 1] : [setDur*1000>=BLU_LngSetDtctMin_Tms && ...
            | setDur*1000 <=BLU_LngSetDtctMax_Tms ]
        (junction 1505) -> (junction 1508) : {send(longSET,Flow);}
        (junction 1503) -> (junction 1510) [order 2]
        (junction 1512) -> (junction 1508)
        (junction 1510) -> (junction 1512)
        ButtonChecker.CANnormal.CheckSet.setHigh -> LongSETRpt [order 2] : after(3,sec)
        (junction 5062) -> (junction 1512)
        LongSETRpt -> (junction 5062) [order 1] : [hasChangedTo(set,false)]
        LongSETRpt -> LongSETRpt [order 2] : [after(BLU_LngBtnEvntDur_Tms,msec)]
      state CheckExit [parallel]
        settings: executionOrder=4
        state exitHigh
          en:
          DASH_RCM_Exit_Switch = eButton.pressed;
          en,du:
          exitDur = elapsed(sec);
        state exitLow
          en:
          DASH_RCM_Exit_Switch = eButton.released;
        (default) -> ButtonChecker.CANnormal.CheckExit.exitLow
        ButtonChecker.CANnormal.CheckExit.exitLow -> ButtonChecker.CANnormal.CheckExit.exitHigh : [hasChangedTo(exitButton,true)]
        (junction 1523) -> ButtonChecker.CANnormal.CheckExit.exitLow
        (junction 1530) -> (junction 1523) : {send(EXIT,Flow);}
        (junction 1517) -> (junction 1530) [order 1] : [exitDur*1000>=BLU_ExitDtctMin_Tms && ...
            | exitDur*1000 <=BLU_ExitDtctMax_Tms ]
        ButtonChecker.CANnormal.CheckExit.exitHigh -> (junction 1517) : [hasChangedTo(exitButton,false)]
        (junction 1534) -> (junction 1523)
        (junction 1517) -> (junction 1521) [order 2]
        (junction 1520) -> (junction 1534) : {send(longEXIT,Flow);}
        (junction 1521) -> (junction 1520) [order 1] : [exitDur*1000>=BLU_LngExitDtctMin_Tms && ...
            | exitDur*1000 <=BLU_LngExitDtctMax_Tms ]
        (junction 1533) -> (junction 1534)
        (junction 1521) -> (junction 1524) [order 2]
        (junction 1524) -> (junction 1533)
      state CheckCC [parallel]
        settings: executionOrder=5
        state setHigh
          en:
          DASH_CC_Master_Switch = eButton.pressed;
          en,du:
          ccDur = elapsed(sec);
        state setLow
          en:
          DASH_CC_Master_Switch = eButton.released;
        (default) -> ButtonChecker.CANnormal.CheckCC.setLow
        (junction 1018) -> ButtonChecker.CANnormal.CheckCC.setHigh : [ECU_CC_Feature==1]
        ButtonChecker.CANnormal.CheckCC.setLow -> (junction 1018) : [hasChangedTo(cc,true)]
        (junction 1001) -> ButtonChecker.CANnormal.CheckCC.setLow : {send(CC,Flow);}
        (junction 1589) -> (junction 1001) : [ccDur >=BLU_CCDtctMin_Tms && ...
            | ccDur <=BLU_CCDtctMax_Tms ]
        ButtonChecker.CANnormal.CheckCC.setHigh -> (junction 1589) : [hasChangedTo(cc,false)]
      state CheckResume [parallel]
        settings: executionOrder=6
        state setHigh
          en:
          DASH_Cc_Resume_Switch = eButton.pressed;
          en,du:
          resumeDur = elapsed(sec)
        state setLow
          en:
          DASH_Cc_Resume_Switch = eButton.released;
        (default) -> ButtonChecker.CANnormal.CheckResume.setLow
        ButtonChecker.CANnormal.CheckResume.setLow -> (junction 1021) : [hasChangedTo(resume,true)]
        (junction 1021) -> ButtonChecker.CANnormal.CheckResume.setHigh : [ECU_CC_Feature==1]
        (junction 1582) -> ButtonChecker.CANnormal.CheckResume.setLow : {send(RESUME,Flow);}
        (junction 1580) -> (junction 1582) : [resumeDur >=BLU_ResDtctMin_Tms && ...
            | resumeDur <=BLU_ResDtctMax_Tms ]
        ButtonChecker.CANnormal.CheckResume.setHigh -> (junction 1580) : [hasChangedTo(resume,false)]
      state CheckCCSet [parallel]
        settings: executionOrder=7
        state setHigh
          en:
          DASH_Cc_Set_Switch = eButton.pressed;
        state setLow
          en:
          DASH_Cc_Set_Switch = eButton.released;
        (default) -> ButtonChecker.CANnormal.CheckCCSet.setLow
        ButtonChecker.CANnormal.CheckCCSet.setLow -> (junction 1038) : [hasChangedTo(ccSet,true)]
        (junction 1038) -> ButtonChecker.CANnormal.CheckCCSet.setHigh : [ECU_CC_Feature==1]
        (junction 1039) -> ButtonChecker.CANnormal.CheckCCSet.setLow [commented out] : {send(longCC,Flow);}
        ButtonChecker.CANnormal.CheckCCSet.setHigh -> (junction 1039) [commented out] : [duration(set==true,msec) >=BLU_LngCCDtctMin_Tms && ...
            | duration(set==true,msec) <=BLU_LngCCDtctMax_Tms ]
        (junction 1040) -> ButtonChecker.CANnormal.CheckCCSet.setLow : [hasChangedTo(cc,false)]
            | {send(CCSet,Flow);}
        ButtonChecker.CANnormal.CheckCCSet.setHigh -> (junction 1040) : [duration(set==true,msec) >=BLU_CCSetDtctMin_Tms && ...
            | duration(set==true,msec) <=BLU_CCSetDtctMax_Tms ]
    state CANerror
      en:
      send(RESET,Flow);
      %currentState = eStateName.CAN_ERROR;
    (default) -> CANnormal
    CANerror -> (junction 4713) [order 2] : [hasChangedTo(up,true)]
    (junction 4713) -> (junction 4715)
    CANnormal -> CANerror : [hasChangedTo(canCommsErr,true)]
    CANerror -> (junction 4715) [order 3] : [hasChangedTo(down,true)]
    (junction 4715) -> (junction 4718)
    CANerror -> CANnormal [order 1] : [hasChangedTo(canCommsErr,false)]
    CANerror -> (junction 4718) [order 4] : [hasChangedTo(set,true)]
    (junction 4718) -> (junction 4721)
    CANerror -> (junction 4721) [order 5] : [hasChangedTo(exitButton,true)]
    (junction 4721) -> (junction 4724)
    (junction 4724) -> CANerror
  state startLapOrLapTimer [function]
    (default) -> (junction 97)
  state copyRoundTimeData [function]
    (default) -> (junction 271) : {index  = 0;}
    (junction 271) -> (junction 274) [order 1] : [index<BLU_MaxRndTimeLoopLim]
    (junction 296) -> (junction 271) : {loc_roundTimerData_arr[index] = roundTimerData_arr[index];}
    (junction 271) -> (junction 278) [order 2]
    (junction 274) -> (junction 296) : {index++;}
  state updateRoundTimeData [function]
    (default) -> (junction 285) : {index  = 0;}
    (junction 285) -> (junction 287) [order 1] : [index<BLU_MaxRndTimeLoopLim]
    (junction 298) -> (junction 285) : { rndTimerData_arr[index]= loc_roundTimerData_arr[index];}
    (junction 285) -> (junction 289) [order 2]
    (junction 287) -> (junction 298) : {index++;}
  data: currState function_input double; currState function_input double; currState function_input double; currState function_input double; currState function_input double; index local double; index local double; set input boolean; up input boolean; down input boolean; DASH_RCM_Set_Switch output Enum: eButton =eButton.released; DASH_RCM_Down_Switch output Enum: eButton =eButton.released; DASH_RCM_Up_Switch output Enum: eButton =eButton.released; DASH_RCM_Exit_Switch output Enum: eButton =eButton.released; exitButton input boolean; ECU_SL_Status parameter; ECU_CC_Feature parameter; DASH_Cc_Resume_Switch output Enum: eButton =eButton.released; DASH_Cc_Set_Switch output Enum: eButton =eButton.released; CCU_RCM_Feature parameter; CCU_RCM_Ready_Status parameter; currentState output Enum: eStateName =eStateName.MAIN_SCREEN; ECU_SL_LED parameter; ECU_SL_Feature parameter; CCU_Status parameter; CCU_TbT_Navigation_Feature parameter; CCU_Music_Feature parameter; Heated_Grips parameter; rideMode output Enum: eRideMode =eRideMode.street; BLU_MaxRndTimeLoopLim parameter; roundTimerData_arr input single size=99; roundTimeCounter output double =0; loc_roundTimerData_arr local single size=99; rndTimerData_arr output single size=99; BLU_UpDtctMax_Tms parameter; BLU_UpDtctMin_Tms parameter; BLU_LngDwnDtctMax_Tms parameter; BLU_LngDwnDtctMin_Tms parameter; BLU_LngExitDtctMax_Tms parameter; BLU_LngExitDtctMin_Tms parameter; BLU_LngSetDtctMax_Tms parameter; BLU_LngSetDtctMin_Tms parameter; ECU_Quickshifter_Feature parameter; BLU_SetDtctMin_Tms parameter; BLU_SetDtctMax_Tms parameter; BLU_LngUpDtctMin_Tms parameter; BLU_LngUpDtctMax_Tms parameter; BLU_ExitDtctMin_Tms parameter; BLU_ExitDtctMax_Tms parameter; BLU_DwnDtctMin_Tms parameter; BLU_DwnDtctMax_Tms parameter; cc input; DASH_CC_Master_Switch output Enum: eButton =eButton.released; BLU_CCDtctMax_Tms parameter; BLU_CCDtctMin_Tms parameter; BLU_ResDtctMax_Tms parameter; BLU_ResDtctMin_Tms parameter; resume input; ccSet input; BLU_CCSetDtctMax_Tms parameter; BLU_CCSetDtctMin_Tms parameter; vehSpeed input; CCU_BT_Status parameter; odoUnits input; exitDur local double; setDur local double; downDur local double; ccDur local double; upDur local double; resumeDur local double; ABS_Launch_Control_Feature parameter; ABS_Mode_Feature parameter; ABS_TC_Mode_Response parameter; ECU_Drive_Mode_Response parameter; DASH_Drive_Mode_Request output uint16 =0; DASH_TC_Mode_Request output uint16 =0; killSwitch input; DASH_ABS_Mode_Request output uint8 =0; ABS_TC_MTC_Mode_Feature parameter; ABS_ABS_Mode_Response parameter; DASH_MTC_Off_Switch output uint16 =0; odoValue input; ShiftLightStatus output uint16 =0; rpm1 output uint16 [5000..11000] =5000; rpm2 output uint16 [5000..11000] =5000; heatedGrips output uint16 =0; quickSelectorDown output uint16 =0; quickSelectorUp output uint16 =0; DASH_RCM_Ready_Switch output uint16; navigationStatus output uint16; QuickSelectorDbg output uint8; HeatedGripsMode local Enum: eHeatedGripsMode =eHeatedGripsMode.OFF; popUpState output uint16; INwarning input; INcall input; INcallEnded input; BLU_BTSearchDur_Tms parameter; btPhoneFound input; jumpDigits local uint8; btPhonePairingError input; btHeadsetPairingError input; btHeadsetFound input; btPhonePassKeyRcvd input; btPhonePrngScs input; phonePaired local boolean =false; headsetPaired local boolean =false; btHdstPrngScs input; DASH_Grip_Installation output uint8 [0..1] =0; HCU_Grip_Heat_Level_Res input; cnfrmAppReg local uint16; rgstrAppReply input; BLU_RND_TMR_LOOP parameter uint8; CCU_Corded_Headset parameter uint8; btPhoneDelErr input; btHdstDelErr input; InCallStatus input; DASH_SL_Switch output; favSlot2Sel local double = eStateName.FAVORITES_TRIP_DISTANCE_1; favSlot3Sel local double =eStateName.FAVORITES_AVERAGE_FUEL_CONS_1; favSlot4Sel local double =eStateName.FAVORITES_TRIP_TIME_1; favSlot1Sel local double =eStateName.FAVORITES_TRIP_DISTANCE_1; IgnBattFlg input; variant parameter; dateFormat local double; canCommsErr input; stateResetFlg input; BLU_LngBtnEvntDur_Tms parameter; BLU_ExtraFnWtTmr_Tms parameter; CCU_Connect parameter
  events: longUP local FUNCTION_CALL_EVENT; UP local FUNCTION_CALL_EVENT; longDOWN local FUNCTION_CALL_EVENT; DOWN local FUNCTION_CALL_EVENT; SET local FUNCTION_CALL_EVENT; EXIT local FUNCTION_CALL_EVENT; longSET local FUNCTION_CALL_EVENT; longEXIT local FUNCTION_CALL_EVENT; CC local FUNCTION_CALL_EVENT; RESUME local FUNCTION_CALL_EVENT; CCSet local FUNCTION_CALL_EVENT; warning local RISING_EDGE_EVENT; incomingCall local RISING_EDGE_EVENT; callEnded local FUNCTION_CALL_EVENT; RESET local FUNCTION_CALL_EVENT
